simulink model "MCVT_Integration"  (R2020a)
settings: Solver=ode8  StartTime=0.0  StopTime=T_end  FixedStep=dt  RelTol=1e-3  EnableMultiTasking=on
values from neighbouring files (symbols this model uses):
  assumed_power_voltage = 1.25e2    (= 125)    [MCVT_Input_File.m]    (also in MCVT_Run_QuickCheck.m)
  battery_power_toggle = 1    [MCVT_Input_File.m]    (also in MCVT_Run_QuickCheck.m)
  battery_trickle_charge_param = 0.1    [MCVT_Input_File.m]    (also in MCVT_Run_QuickCheck.m)
  battery_trickle_leakage_param = 0.01    [MCVT_Input_File.m]    (also in MCVT_Run_QuickCheck.m)
  damage_case_id = 3    [MCVT_Input_File.m]    (also in MCVT_Run_QuickCheck.m = 1)
  dt = 1/fs    (= 0.001)    [MCVT_Run_QuickCheck.m]    (also in MCVT_Sim_Set.m)
  dt_2 = 1/fs_2    (= 0.001)    [MCVT_Run_QuickCheck.m]    (also in MCVT_Sim_Set.m)
  dt_3 = 1/fs_3    (= 0.001)    [MCVT_Run_QuickCheck.m]    (also in MCVT_Sim_Set.m)
  dt_5 = 1/fs_5    (= 0.005)    [MCVT_Run_QuickCheck.m]    (also in MCVT_Sim_Set.m)
  dt_8 = 1/fs_8    (= 0.05)    [MCVT_Run_QuickCheck.m]    (also in MCVT_Sim_Set.m)
  dt_9 = 1/fs_9    (= 0.001)    [MCVT_Run_QuickCheck.m]    (also in MCVT_Sim_Set.m)
  dust_met_duration = 3    [MCVT_Input_File.m]    (also in MCVT_Run_QuickCheck.m = 2)
  ECLSS_indoor_set_temperature = 298.15    [MCVT_Input_File.m]    (also in MCVT_Run_QuickCheck.m)
  energy_store_max_o = 150    [MCVT_Input_File.m]    (also in MCVT_Run_QuickCheck.m)
  energy_store_o = 120    [MCVT_Input_File.m]    (also in MCVT_Run_QuickCheck.m)
  fs = 1000    [MCVT_Run_QuickCheck.m]    (also in MCVT_Sim_Set.m)
  gain_charge_param = 1    [MCVT_Input_File.m]    (also in MCVT_Run_QuickCheck.m)
  ground_temperature = 373    [MCVT_Habitat_Design_Input_File.m]    (also in MCVT_Run_QuickCheck.m)
  I_max_charge = 800    [MCVT_Input_File.m]    (also in MCVT_Run_QuickCheck.m)
  I_max_discharge = 800    [MCVT_Input_File.m]    (also in MCVT_Run_QuickCheck.m)
  initial_pressure = 1e5    (= 100000)    [MCVT_Input_File.m]    (also in MCVT_Run_QuickCheck.m)
  initial_temperature = 295    [MCVT_Input_File.m]    (also in MCVT_Run_QuickCheck.m)
  interior_leak_rate = 0.00415    [MCVT_Input_File.m]    (also in MCVT_Run_QuickCheck.m)
  launch_dust_accum_start_time = 10    [MCVT_Input_File.m]    (also in MCVT_Run_QuickCheck.m = 2)
  launch_dust_accum_stop_time = 12    [MCVT_Input_File.m]    (also in MCVT_Run_QuickCheck.m = 3)
  meteorite_impact_time = 2    [MCVT_Input_File.m]    (also in MCVT_Run_QuickCheck.m = 1)
  nuclear_power_toggle = 1    [MCVT_Input_File.m]    (also in MCVT_Run_QuickCheck.m)
  print_interval = 30    [MCVT_Input_File.m]
  SOC_lower_thres = 0.5    [MCVT_Input_File.m]    (also in MCVT_Run_QuickCheck.m)
  SOC_upper_thres = 0.9    [MCVT_Input_File.m]    (also in MCVT_Run_QuickCheck.m)
  solar_power_toggle = 1    [MCVT_Input_File.m]    (also in MCVT_Run_QuickCheck.m)
  space_temperature = 4    [MCVT_Habitat_Design_Input_File.m]    (also in MCVT_Run_QuickCheck.m)
  Start_angle = 90    [MCVT_Input_File.m]    (also in MCVT_Run_QuickCheck.m)
undefined symbols (not defined in the file or in its folder): A_flow_restrictor, ACTIVITY_LOOKUP_TABLE, air, Aref, Bmap, Bmap2, Bmap3, comms, DiscIntNeverNeededParam, DmgSPL, dust_parameters, ECLSS_dust_damage_repair_time, ECLSS_heater_capacity, ECLSS_IPS_restrictor, ECLSS_IPS_setpointPressure, ECLSS_paint_damage_repair_time, ECLSS_radiatorLoop_discreteNumber, ECLSS_Vst, emptyActivity, expected_heat_add, FI, habitat, HIEM2SMM, HIEM2STM, HIEMDamageElements, impact_numbering, initial_SPLT_damage_index, input_air, input_Cond, input_CP, input_discretization, input_EV, input_Evap, input_radiatorLoop, K_ECLSS_dust_single, K_ECLSS_paint_single, K_Structure_damage_single, Kref, kW_to_W, meteoroidDustDep, mFRF, ndelays, nominalDustDep, noverlap, nuclear_toggle_gain, output_limits, panel_paint0, Ps0, reference_temperature, RepairTime_ECLSS_factor, Sensor_Location_SMM, SMM_T0, SMM_T1, SMM_T2, SMM_T3, SMM_T4, SMM_T4_0, SMM_T4_1, SMM_T4_2, solar_FDD_K ... (+73)
libraries: neural x350, simulink x39, xpcutilitieslib x7, xpcrtudplib x7, dspstat3 x1
(part 36 of 43)

== system MCVT_Integration/Sys3 - Power System/Power Generation Subsystem1/Nuclear Power Generation/SP-100 Power Generation/	Power Generation/Sensor Models/Thermocouple Model Stub/Omega XMO S-type Thermocouple ==
  Actual Temperature : Inport
  Kelvin to Celcuis : Bias  Bias=-273.15
  Thermocouple model 7 : Lookup_n-D  NumberOfTableDimensions=1  BreakpointsForDimension1=TypeS_degC  BeginIndexSearchUsingPreviousIndexResult=on  Table=TypeS_Volts  DiagnosticForOutOfRangeInput=Warning  InputSameDT=on
      settings: AttributesFormatString=%<bp1> to\n%<tableData>
  microvolts : Outport
  wires:
  Kelvin to Celcuis/1 -> Thermocouple model 7/1
  Actual Temperature/1 -> Kelvin to Celcuis/1
  Thermocouple model 7/1 -> microvolts/1

== system MCVT_Integration/Sys3 - Power System/Power Generation Subsystem1/Nuclear Power Generation/SP-100 Power Generation/	Power Generation/Sensor Models/Thermocouple Model2 Stub/MAX31856 Precision Thermocouple to Digital Converter with Linearization  ==
  Thermocouple Measurement : Inport
  External Cold Junction Measurement : Inport  Port=2
  Celcuis to Kelvin : Bias  Bias=273.15
  Thermocouple conditioning 7 : Lookup_n-D  NumberOfTableDimensions=1  BreakpointsForDimension1=TypeS_Volts  BeginIndexSearchUsingPreviousIndexResult=on  Table=TypeS_degC  DiagnosticForOutOfRangeInput=Warning  InputSameDT=on
      settings: AttributesFormatString=%<bp1> to\n%<tableData>
  Estimated Process Temperature : Outport
  Cyber Output : Outport  Port=2
  wires:
  Thermocouple conditioning 7/1 -> Celcuis to Kelvin/1
  Thermocouple Measurement/1 -> Thermocouple conditioning 7/1
  Celcuis to Kelvin/1 -> Estimated Process Temperature/1

== system MCVT_Integration/Sys3 - Power System/Power Generation Subsystem1/Nuclear Power Generation/SP-100 Power Generation/	Power Generation/Sensor Models/Thermocouple Model2 Stub/Omega XMO S-type Thermocouple ==
  Actual Temperature : Inport
  Kelvin to Celcuis : Bias  Bias=-273.15
  Thermocouple model 7 : Lookup_n-D  NumberOfTableDimensions=1  BreakpointsForDimension1=TypeS_degC  BeginIndexSearchUsingPreviousIndexResult=on  Table=TypeS_Volts  DiagnosticForOutOfRangeInput=Warning  InputSameDT=on
      settings: AttributesFormatString=%<bp1> to\n%<tableData>
  microvolts : Outport
  wires:
  Kelvin to Celcuis/1 -> Thermocouple model 7/1  "temp_test"
  Actual Temperature/1 -> Kelvin to Celcuis/1
  Thermocouple model 7/1 -> microvolts/1

== system MCVT_Integration/Sys3 - Power System/Power Generation Subsystem1/Nuclear Power Generation/SP-100 Power Generation/	Power Generation/Sensor Models/Thermocouple Model3 Stub/MAX31856 Precision Thermocouple to Digital Converter with Linearization  ==
  Thermocouple Measurement : Inport
  External Cold Junction Measurement : Inport  Port=2
  Celcuis to Kelvin : Bias  Bias=273.15
  Thermocouple conditioning 7 : Lookup_n-D  NumberOfTableDimensions=1  BreakpointsForDimension1=TypeS_Volts  BeginIndexSearchUsingPreviousIndexResult=on  Table=TypeS_degC  DiagnosticForOutOfRangeInput=Warning  InputSameDT=on
      settings: AttributesFormatString=%<bp1> to\n%<tableData>
  Estimated Process Temperature : Outport
  Cyber Output : Outport  Port=2
  wires:
  Thermocouple Measurement/1 -> Thermocouple conditioning 7/1
  Thermocouple conditioning 7/1 -> Celcuis to Kelvin/1
  Celcuis to Kelvin/1 -> Estimated Process Temperature/1

== system MCVT_Integration/Sys3 - Power System/Power Generation Subsystem1/Nuclear Power Generation/SP-100 Power Generation/	Power Generation/Sensor Models/Thermocouple Model3 Stub/Omega XMO S-type Thermocouple ==
  Actual Temperature : Inport
  Kelvin to Celcuis : Bias  Bias=-273.15
  Thermocouple model 7 : Lookup_n-D  NumberOfTableDimensions=1  BreakpointsForDimension1=TypeS_degC  BeginIndexSearchUsingPreviousIndexResult=on  Table=TypeS_Volts  DiagnosticForOutOfRangeInput=Warning  InputSameDT=on
      settings: AttributesFormatString=%<bp1> to\n%<tableData>
  microvolts : Outport
  wires:
  Kelvin to Celcuis/1 -> Thermocouple model 7/1
  Actual Temperature/1 -> Kelvin to Celcuis/1
  Thermocouple model 7/1 -> microvolts/1

== system MCVT_Integration/Sys3 - Power System/Power Generation Subsystem1/Nuclear Power Generation/SP-100 Power Generation/	Power Generation/Sensor Models/Thermocouple Model4 Stub/MAX31856 Precision Thermocouple to Digital Converter with Linearization  ==
  Thermocouple Measurement : Inport
  External Cold Junction Measurement : Inport  Port=2
  Celcuis to Kelvin : Bias  Bias=273.15
  Thermocouple conditioning 7 : Lookup_n-D  NumberOfTableDimensions=1  BreakpointsForDimension1=TypeS_Volts  BeginIndexSearchUsingPreviousIndexResult=on  Table=TypeS_degC  DiagnosticForOutOfRangeInput=Warning  InputSameDT=on
      settings: AttributesFormatString=%<bp1> to\n%<tableData>
  Estimated Process Temperature : Outport
  Cyber Output : Outport  Port=2
  wires:
  Thermocouple conditioning 7/1 -> Celcuis to Kelvin/1
  Thermocouple Measurement/1 -> Thermocouple conditioning 7/1
  Celcuis to Kelvin/1 -> Estimated Process Temperature/1

== system MCVT_Integration/Sys3 - Power System/Power Generation Subsystem1/Nuclear Power Generation/SP-100 Power Generation/	Power Generation/Sensor Models/Thermocouple Model4 Stub/Omega XMO S-type Thermocouple ==
  Actual Temperature : Inport
  Kelvin to Celcuis : Bias  Bias=-273.15
  Thermocouple model 7 : Lookup_n-D  NumberOfTableDimensions=1  BreakpointsForDimension1=TypeS_degC  BeginIndexSearchUsingPreviousIndexResult=on  Table=TypeS_Volts  DiagnosticForOutOfRangeInput=Warning  InputSameDT=on
      settings: AttributesFormatString=%<bp1> to\n%<tableData>
  microvolts : Outport
  wires:
  Kelvin to Celcuis/1 -> Thermocouple model 7/1
  Actual Temperature/1 -> Kelvin to Celcuis/1
  Thermocouple model 7/1 -> microvolts/1

== system MCVT_Integration/Sys3 - Power System/Power Generation Subsystem1/Nuclear Power Generation/SP-100 Power Generation/FDD Iterative Online Residual Analysis1/ Digital Twin of SP100/Residual Generation/Compare to Nominal ==
  Physical Output SP100 : Inport
  power nominal : Inport  Port=2
  Sub3_In_Intervention : Inport  Port=3
  CUSUM5 : Subsystem
  Mux5 : Mux  Inputs=3
  Subtract10 : Sum  Inputs=-+
  Terminator5 : Terminator
  sub3_cusum_sigma : Constant  Value=sub3_cusum_sigma
  FDD : Outport
  wires:
  [nom_power] -> Subtract10/2  "power_nominal (kW)"
  Physical Output SP100/1 -> [pow_actual]  "pow_actual (kW)"
  Subtract10/1 -> [power_resids]  "Power Residuals Compare"
  [sigma] -> CUSUM5/2(sigma)
  CUSUM5/1(Bias Indication) -> Mux5/1, [FDD_signal]  "Pow_bias"
  CUSUM5/2(Positive Bias Indication) -> Mux5/2  "Pow_Pos_bias"
  CUSUM5/3(Negative Bias Indication) -> Mux5/3  "Pow_Neg_bias"
  Mux5/1 -> Terminator5/1  "Power_indicators_compare"
  [fdd_int] -> CUSUM5/3(Sub3_In_Intervention)
  [FDD_signal] -> FDD/1
  [pow_actual] -> Subtract10/1
  power nominal/1 -> [nom_power]  "power_nominal (kW)"
  Sub3_In_Intervention/1 -> [fdd_int]
  [power_resids] -> CUSUM5/1(residual)
  sub3_cusum_sigma/1 -> [sigma]

== system MCVT_Integration/Sys3 - Power System/Power Generation Subsystem1/Nuclear Power Generation/SP-100 Power Generation/	Power Generation/Nominal Power/Sensor Models/Cold Junction Temperature Sensing Model/ Resistance Temperature Detector ==
  Physical Interdependency - IN : Inport
  Switch Control : Inport  Port=2
  R = U/I : Gain  Gain=1/0.001
  R vs T : MATLAB Function  TreatAsAtomicUnit=on
  RTD with Self-heat Error : Subsystem
  RTD without Self-heat Error : Subsystem
  Self-heat Calculation : Subsystem
  Switch ON/OFF Control Block : Subsystem
  T(Celcuis) vs R : MATLAB Function  TreatAsAtomicUnit=on
  Read Temperature : Outport  VectorParamsAs1DForOutWhenUnconnected=off
  Measured Temperature : Outport  Port=2  VectorParamsAs1DForOutWhenUnconnected=off
  wires:
  T(Celcuis) vs R/1(R) -> RTD without Self-heat Error/1(Resistance Change without Error), Self-heat Calculation/2(Resistance Change without Error)
  R vs T/1(T) -> Measured Temperature/1
  RTD with Self-heat Error/1(Voltage Output with Error) -> R = U/I/1
  Self-heat Calculation/1(Resistance Change with Error) -> RTD with Self-heat Error/1(Resistance Change with Error)
  R = U/I/1 -> R vs T/1(R)
  RTD without Self-heat Error/1(Current in Circuit) -> Self-heat Calculation/3(Current in Circuit)
  Switch ON/OFF Control Block/1(Out1) -> T(Celcuis) vs R/1(T), Self-heat Calculation/1(Real Temperature)
  Switch Control/1 -> Switch ON/OFF Control Block/1(In1)
  Physical Interdependency - IN/1 -> Read Temperature/1, Switch ON/OFF Control Block/2(In2)

== system MCVT_Integration/Sys3 - Power System/Power Generation Subsystem1/Nuclear Power Generation/SP-100 Power Generation/	Power Generation/Nominal Power/Sensor Models/Cold Junction Temperature Sensing Model1/ Resistance Temperature Detector ==
  Physical Interdependency - IN : Inport
  Switch Control : Inport  Port=2
  R = U/I : Gain  Gain=1/0.001
  R vs T : MATLAB Function  TreatAsAtomicUnit=on
  RTD with Self-heat Error : Subsystem
  RTD without Self-heat Error : Subsystem
  Self-heat Calculation : Subsystem
  Switch ON/OFF Control Block : Subsystem
  T(Celcuis) vs R : MATLAB Function  TreatAsAtomicUnit=on
  Temperature Output with Error : Scope
  Read Temperature : Outport  VectorParamsAs1DForOutWhenUnconnected=off
  Measured Temperature : Outport  Port=2  VectorParamsAs1DForOutWhenUnconnected=off
  wires:
  T(Celcuis) vs R/1(R) -> RTD without Self-heat Error/1(Resistance Change without Error), Self-heat Calculation/2(Resistance Change without Error)
  R vs T/1(T) -> Measured Temperature/1, Temperature Output with Error/1
  RTD with Self-heat Error/1(Voltage Output with Error) -> R = U/I/1
  Self-heat Calculation/1(Resistance Change with Error) -> RTD with Self-heat Error/1(Resistance Change with Error)
  R = U/I/1 -> R vs T/1(R)
  RTD without Self-heat Error/1(Current in Circuit) -> Self-heat Calculation/3(Current in Circuit)
  Switch ON/OFF Control Block/1(Out1) -> T(Celcuis) vs R/1(T), Self-heat Calculation/1(Real Temperature)
  Switch Control/1 -> Switch ON/OFF Control Block/1(In1)
  Physical Interdependency - IN/1 -> Read Temperature/1, Switch ON/OFF Control Block/2(In2)

== system MCVT_Integration/Sys3 - Power System/Power Generation Subsystem1/Nuclear Power Generation/SP-100 Power Generation/	Power Generation/Nominal Power/Sensor Models/Cold Junction Temperature Sensing Model2/ Resistance Temperature Detector ==
  Physical Interdependency - IN : Inport
  Switch Control : Inport  Port=2
  R = U/I : Gain  Gain=1/0.001
  R vs T : MATLAB Function  TreatAsAtomicUnit=on
  RTD with Self-heat Error : Subsystem
  RTD without Self-heat Error : Subsystem
  Self-heat Calculation : Subsystem
  Switch ON/OFF Control Block : Subsystem
  T(Celcuis) vs R : MATLAB Function  TreatAsAtomicUnit=on
  Temperature Output with Error : Scope
  Read Temperature : Outport  VectorParamsAs1DForOutWhenUnconnected=off
  Measured Temperature : Outport  Port=2  VectorParamsAs1DForOutWhenUnconnected=off
  wires:
  Physical Interdependency - IN/1 -> Switch ON/OFF Control Block/2(In2), Read Temperature/1
  Switch Control/1 -> Switch ON/OFF Control Block/1(In1)
  Switch ON/OFF Control Block/1(Out1) -> Self-heat Calculation/1(Real Temperature), T(Celcuis) vs R/1(T)
  RTD without Self-heat Error/1(Current in Circuit) -> Self-heat Calculation/3(Current in Circuit)
  R = U/I/1 -> R vs T/1(R)
  Self-heat Calculation/1(Resistance Change with Error) -> RTD with Self-heat Error/1(Resistance Change with Error)
  RTD with Self-heat Error/1(Voltage Output with Error) -> R = U/I/1
  R vs T/1(T) -> Temperature Output with Error/1, Measured Temperature/1
  T(Celcuis) vs R/1(R) -> Self-heat Calculation/2(Resistance Change without Error), RTD without Self-heat Error/1(Resistance Change without Error)

== system MCVT_Integration/Sys3 - Power System/Power Generation Subsystem1/Nuclear Power Generation/SP-100 Power Generation/	Power Generation/Nominal Power/Sensor Models/Cold Junction Temperature Sensing Model3/ Resistance Temperature Detector ==
  Physical Interdependency - IN : Inport
  Switch Control : Inport  Port=2
  R = U/I : Gain  Gain=1/0.001
  R vs T : MATLAB Function  TreatAsAtomicUnit=on
  RTD with Self-heat Error : Subsystem
  RTD without Self-heat Error : Subsystem
  Self-heat Calculation : Subsystem
  Switch ON/OFF Control Block : Subsystem
  T(Celcuis) vs R : MATLAB Function  TreatAsAtomicUnit=on
  Temperature Output with Error : Scope
  Read Temperature : Outport  VectorParamsAs1DForOutWhenUnconnected=off
  Measured Temperature : Outport  Port=2  VectorParamsAs1DForOutWhenUnconnected=off
  wires:
  T(Celcuis) vs R/1(R) -> RTD without Self-heat Error/1(Resistance Change without Error), Self-heat Calculation/2(Resistance Change without Error)
  R vs T/1(T) -> Measured Temperature/1, Temperature Output with Error/1
  RTD with Self-heat Error/1(Voltage Output with Error) -> R = U/I/1
  Self-heat Calculation/1(Resistance Change with Error) -> RTD with Self-heat Error/1(Resistance Change with Error)
  R = U/I/1 -> R vs T/1(R)
  RTD without Self-heat Error/1(Current in Circuit) -> Self-heat Calculation/3(Current in Circuit)
  Switch ON/OFF Control Block/1(Out1) -> T(Celcuis) vs R/1(T), Self-heat Calculation/1(Real Temperature)
  Switch Control/1 -> Switch ON/OFF Control Block/1(In1)
  Physical Interdependency - IN/1 -> Read Temperature/1, Switch ON/OFF Control Block/2(In2)

== system MCVT_Integration/Sys3 - Power System/Power Generation Subsystem1/Nuclear Power Generation/SP-100 Power Generation/	Power Generation/Nominal Power/Sensor Models/Cold Junction Temperature Sensing Model4/ Resistance Temperature Detector ==
  Physical Interdependency - IN : Inport
  Switch Control : Inport  Port=2
  R = U/I : Gain  Gain=1/0.001
  R vs T : MATLAB Function  TreatAsAtomicUnit=on
  RTD with Self-heat Error : Subsystem
  RTD without Self-heat Error : Subsystem
  Self-heat Calculation : Subsystem
  Switch ON/OFF Control Block : Subsystem
  T(Celcuis) vs R : MATLAB Function  TreatAsAtomicUnit=on
  Temperature Output with Error : Scope
  Read Temperature : Outport  VectorParamsAs1DForOutWhenUnconnected=off
  Measured Temperature : Outport  Port=2  VectorParamsAs1DForOutWhenUnconnected=off
  wires:
  Physical Interdependency - IN/1 -> Switch ON/OFF Control Block/2(In2), Read Temperature/1
  Switch Control/1 -> Switch ON/OFF Control Block/1(In1)
  Switch ON/OFF Control Block/1(Out1) -> Self-heat Calculation/1(Real Temperature), T(Celcuis) vs R/1(T)
  RTD without Self-heat Error/1(Current in Circuit) -> Self-heat Calculation/3(Current in Circuit)
  R = U/I/1 -> R vs T/1(R)
  Self-heat Calculation/1(Resistance Change with Error) -> RTD with Self-heat Error/1(Resistance Change with Error)
  RTD with Self-heat Error/1(Voltage Output with Error) -> R = U/I/1
  R vs T/1(T) -> Temperature Output with Error/1, Measured Temperature/1
  T(Celcuis) vs R/1(R) -> Self-heat Calculation/2(Resistance Change without Error), RTD without Self-heat Error/1(Resistance Change without Error)

== system MCVT_Integration/Sys3 - Power System/Power Generation Subsystem1/Nuclear Power Generation/SP-100 Power Generation/	Power Generation/Nominal Power/Sensor Models/Thermocouple Mode Stub/MAX31856 Precision Thermocouple to Digital Converter with Linearization  ==
  Thermocouple Measurement : Inport
  External Cold Junction Measurement : Inport  Port=2
  Celcuis to Kelvin : Bias  Bias=273.15
  Thermocouple conditioning 7 : Lookup_n-D  NumberOfTableDimensions=1  BreakpointsForDimension1=TypeS_Volts  BeginIndexSearchUsingPreviousIndexResult=on  Table=TypeS_degC  DiagnosticForOutOfRangeInput=Warning  InputSameDT=on
      settings: AttributesFormatString=%<bp1> to\n%<tableData>
  Estimated Process Temperature : Outport
  Cyber Output : Outport  Port=2
  wires:
  Thermocouple conditioning 7/1 -> Celcuis to Kelvin/1
  Thermocouple Measurement/1 -> Thermocouple conditioning 7/1
  Celcuis to Kelvin/1 -> Estimated Process Temperature/1

== system MCVT_Integration/Sys3 - Power System/Power Generation Subsystem1/Nuclear Power Generation/SP-100 Power Generation/	Power Generation/Nominal Power/Sensor Models/Thermocouple Mode Stub/Omega XMO S-type Thermocouple ==
  Actual Temperature : Inport
  Kelvin to Celcuis : Bias  Bias=-273.15
  Thermocouple model 7 : Lookup_n-D  NumberOfTableDimensions=1  BreakpointsForDimension1=TypeS_degC  BeginIndexSearchUsingPreviousIndexResult=on  Table=TypeS_Volts  DiagnosticForOutOfRangeInput=Warning  InputSameDT=on
      settings: AttributesFormatString=%<bp1> to\n%<tableData>
  microvolts : Outport
  wires:
  Actual Temperature/1 -> Kelvin to Celcuis/1
  Kelvin to Celcuis/1 -> Thermocouple model 7/1
  Thermocouple model 7/1 -> microvolts/1

== system MCVT_Integration/Sys3 - Power System/Power Generation Subsystem1/Nuclear Power Generation/SP-100 Power Generation/	Power Generation/Nominal Power/Sensor Models/Thermocouple Model Stub/MAX31856 Precision Thermocouple to Digital Converter with Linearization  ==
  Thermocouple Measurement : Inport
  External Cold Junction Measurement : Inport  Port=2
  Celcuis to Kelvin : Bias  Bias=273.15
  Thermocouple conditioning 7 : Lookup_n-D  NumberOfTableDimensions=1  BreakpointsForDimension1=TypeS_Volts  BeginIndexSearchUsingPreviousIndexResult=on  Table=TypeS_degC  DiagnosticForOutOfRangeInput=Warning  InputSameDT=on
      settings: AttributesFormatString=%<bp1> to\n%<tableData>
  Estimated Process Temperature : Outport
  Cyber Output : Outport  Port=2
  wires:
  Thermocouple conditioning 7/1 -> Celcuis to Kelvin/1
  Thermocouple Measurement/1 -> Thermocouple conditioning 7/1
  Celcuis to Kelvin/1 -> Estimated Process Temperature/1

== system MCVT_Integration/Sys3 - Power System/Power Generation Subsystem1/Nuclear Power Generation/SP-100 Power Generation/	Power Generation/Nominal Power/Sensor Models/Thermocouple Model Stub/Omega XMO S-type Thermocouple ==
  Actual Temperature : Inport
  Kelvin to Celcuis : Bias  Bias=-273.15
  Thermocouple model 7 : Lookup_n-D  NumberOfTableDimensions=1  BreakpointsForDimension1=TypeS_degC  BeginIndexSearchUsingPreviousIndexResult=on  Table=TypeS_Volts  DiagnosticForOutOfRangeInput=Warning  InputSameDT=on
      settings: AttributesFormatString=%<bp1> to\n%<tableData>
  microvolts : Outport
  wires:
  Kelvin to Celcuis/1 -> Thermocouple model 7/1
  Actual Temperature/1 -> Kelvin to Celcuis/1
  Thermocouple model 7/1 -> microvolts/1

== system MCVT_Integration/Sys3 - Power System/Power Generation Subsystem1/Nuclear Power Generation/SP-100 Power Generation/	Power Generation/Nominal Power/Sensor Models/Thermocouple Model2 Stub/MAX31856 Precision Thermocouple to Digital Converter with Linearization  ==
  Thermocouple Measurement : Inport
  External Cold Junction Measurement : Inport  Port=2
  Celcuis to Kelvin : Bias  Bias=273.15
  Thermocouple conditioning 7 : Lookup_n-D  NumberOfTableDimensions=1  BreakpointsForDimension1=TypeS_Volts  BeginIndexSearchUsingPreviousIndexResult=on  Table=TypeS_degC  DiagnosticForOutOfRangeInput=Warning  InputSameDT=on
      settings: AttributesFormatString=%<bp1> to\n%<tableData>
  Estimated Process Temperature : Outport
  Cyber Output : Outport  Port=2
  wires:
  Thermocouple conditioning 7/1 -> Celcuis to Kelvin/1
  Thermocouple Measurement/1 -> Thermocouple conditioning 7/1
  Celcuis to Kelvin/1 -> Estimated Process Temperature/1

== system MCVT_Integration/Sys3 - Power System/Power Generation Subsystem1/Nuclear Power Generation/SP-100 Power Generation/	Power Generation/Nominal Power/Sensor Models/Thermocouple Model2 Stub/Omega XMO S-type Thermocouple ==
  Actual Temperature : Inport
  Kelvin to Celcuis : Bias  Bias=-273.15
  Thermocouple model 7 : Lookup_n-D  NumberOfTableDimensions=1  BreakpointsForDimension1=TypeS_degC  BeginIndexSearchUsingPreviousIndexResult=on  Table=TypeS_Volts  DiagnosticForOutOfRangeInput=Warning  InputSameDT=on
      settings: AttributesFormatString=%<bp1> to\n%<tableData>
  microvolts : Outport
  wires:
  Kelvin to Celcuis/1 -> Thermocouple model 7/1  "temp_test"
  Actual Temperature/1 -> Kelvin to Celcuis/1
  Thermocouple model 7/1 -> microvolts/1

== system MCVT_Integration/Sys3 - Power System/Power Generation Subsystem1/Nuclear Power Generation/SP-100 Power Generation/	Power Generation/Nominal Power/Sensor Models/Thermocouple Model3 Stub/MAX31856 Precision Thermocouple to Digital Converter with Linearization  ==
  Thermocouple Measurement : Inport
  External Cold Junction Measurement : Inport  Port=2
  Celcuis to Kelvin : Bias  Bias=273.15
  Thermocouple conditioning 7 : Lookup_n-D  NumberOfTableDimensions=1  BreakpointsForDimension1=TypeS_Volts  BeginIndexSearchUsingPreviousIndexResult=on  Table=TypeS_degC  DiagnosticForOutOfRangeInput=Warning  InputSameDT=on
      settings: AttributesFormatString=%<bp1> to\n%<tableData>
  Estimated Process Temperature : Outport
  Cyber Output : Outport  Port=2
  wires:
  Thermocouple Measurement/1 -> Thermocouple conditioning 7/1
  Thermocouple conditioning 7/1 -> Celcuis to Kelvin/1
  Celcuis to Kelvin/1 -> Estimated Process Temperature/1

== system MCVT_Integration/Sys3 - Power System/Power Generation Subsystem1/Nuclear Power Generation/SP-100 Power Generation/	Power Generation/Nominal Power/Sensor Models/Thermocouple Model3 Stub/Omega XMO S-type Thermocouple ==
  Actual Temperature : Inport
  Kelvin to Celcuis : Bias  Bias=-273.15
  Thermocouple model 7 : Lookup_n-D  NumberOfTableDimensions=1  BreakpointsForDimension1=TypeS_degC  BeginIndexSearchUsingPreviousIndexResult=on  Table=TypeS_Volts  DiagnosticForOutOfRangeInput=Warning  InputSameDT=on
      settings: AttributesFormatString=%<bp1> to\n%<tableData>
  microvolts : Outport
  wires:
  Kelvin to Celcuis/1 -> Thermocouple model 7/1
  Actual Temperature/1 -> Kelvin to Celcuis/1
  Thermocouple model 7/1 -> microvolts/1

== system MCVT_Integration/Sys3 - Power System/Power Generation Subsystem1/Nuclear Power Generation/SP-100 Power Generation/	Power Generation/Nominal Power/Sensor Models/Thermocouple Model4 Stub/MAX31856 Precision Thermocouple to Digital Converter with Linearization  ==
  Thermocouple Measurement : Inport
  External Cold Junction Measurement : Inport  Port=2
  Celcuis to Kelvin : Bias  Bias=273.15
  Thermocouple conditioning 7 : Lookup_n-D  NumberOfTableDimensions=1  BreakpointsForDimension1=TypeS_Volts  BeginIndexSearchUsingPreviousIndexResult=on  Table=TypeS_degC  DiagnosticForOutOfRangeInput=Warning  InputSameDT=on
      settings: AttributesFormatString=%<bp1> to\n%<tableData>
  Estimated Process Temperature : Outport
  Cyber Output : Outport  Port=2
  wires:
  Thermocouple conditioning 7/1 -> Celcuis to Kelvin/1
  Thermocouple Measurement/1 -> Thermocouple conditioning 7/1
  Celcuis to Kelvin/1 -> Estimated Process Temperature/1

== system MCVT_Integration/Sys3 - Power System/Power Generation Subsystem1/Nuclear Power Generation/SP-100 Power Generation/	Power Generation/Nominal Power/Sensor Models/Thermocouple Model4 Stub/Omega XMO S-type Thermocouple ==
  Actual Temperature : Inport
  Kelvin to Celcuis : Bias  Bias=-273.15
  Thermocouple model 7 : Lookup_n-D  NumberOfTableDimensions=1  BreakpointsForDimension1=TypeS_degC  BeginIndexSearchUsingPreviousIndexResult=on  Table=TypeS_Volts  DiagnosticForOutOfRangeInput=Warning  InputSameDT=on
      settings: AttributesFormatString=%<bp1> to\n%<tableData>
  microvolts : Outport
  wires:
  Kelvin to Celcuis/1 -> Thermocouple model 7/1
  Actual Temperature/1 -> Kelvin to Celcuis/1
  Thermocouple model 7/1 -> microvolts/1

== system MCVT_Integration/Sys3 - Power System/Power Generation Subsystem1/Nuclear Power Generation/SP-100 Power Generation/	Power Generation/Sensor Models/Cold Junction Temperature Sensing Model/ Resistance Temperature Detector/RTD with Self-heat Error ==
  Resistance Change with Error : Inport
  " Current in Circuit" : Constant  Value=0.001
  MATLAB Function : MATLAB Function  TreatAsAtomicUnit=on
  Voltage Output with Error : Outport  VectorParamsAs1DForOutWhenUnconnected=off
  wires:
  Resistance Change with Error/1 -> MATLAB Function/2(R)
  MATLAB Function/1(U) -> Voltage Output with Error/1
  " Current in Circuit"/1 -> MATLAB Function/1(I)

== system MCVT_Integration/Sys3 - Power System/Power Generation Subsystem1/Nuclear Power Generation/SP-100 Power Generation/	Power Generation/Sensor Models/Cold Junction Temperature Sensing Model/ Resistance Temperature Detector/RTD without Self-heat Error ==
  Resistance Change without Error : Inport
  " Current in Circuit" : Constant  Value=0.001
  MATLAB Function : MATLAB Function  TreatAsAtomicUnit=on
  Current in Circuit : Outport  VectorParamsAs1DForOutWhenUnconnected=off
  Voltage Output without Error : Outport  Port=2  VectorParamsAs1DForOutWhenUnconnected=off
  wires:
  Resistance Change without Error/1 -> MATLAB Function/2(R)
  MATLAB Function/2(U) -> Voltage Output without Error/1
  MATLAB Function/1(I) -> Current in Circuit/1
  " Current in Circuit"/1 -> MATLAB Function/1("I ")

== system MCVT_Integration/Sys3 - Power System/Power Generation Subsystem1/Nuclear Power Generation/SP-100 Power Generation/	Power Generation/Sensor Models/Cold Junction Temperature Sensing Model/ Resistance Temperature Detector/Self-heat Calculation ==
  note: Power = I 2 *R
  Real Temperature : Inport
  Resistance Change without Error : Inport  Port=2
  Current in Circuit : Inport  Port=3
  "  T vs R " : MATLAB Function  TreatAsAtomicUnit=on
  Add1 : Sum
  Divide1 : Product  Inputs=*/
  Gain : Gain  Gain=1000
  Product : Product
  Self Heating Constant (mW/℃) : Constant  Value=15
  Square : Math  Operator=square  SignedPower=on  SaturateOnIntegerOverflow=on
  Resistance Change with Error : Outport  VectorParamsAs1DForOutWhenUnconnected=off
  Self-heat Error : Outport  Port=2  VectorParamsAs1DForOutWhenUnconnected=off
  wires:
  Self Heating Constant (mW/℃)/1 -> Divide1/2
  Product/1 -> Divide1/1
  Add1/1 -> "  T vs R "/1(T)
  Divide1/1 -> Self-heat Error/1, Add1/2
  Resistance Change without Error/1 -> Product/1
  "  T vs R "/1(R) -> Resistance Change with Error/1
  Real Temperature/1 -> Add1/1
  Gain/1 -> Square/1
  Square/1 -> Product/2
  Current in Circuit/1 -> Gain/1

== system MCVT_Integration/Sys3 - Power System/Power Generation Subsystem1/Nuclear Power Generation/SP-100 Power Generation/	Power Generation/Sensor Models/Cold Junction Temperature Sensing Model/ Resistance Temperature Detector/Switch ON//OFF Control Block ==
  In1 : Inport
  In2 : Inport  Port=2
  Multiport Switch1 : MultiPortSwitch  Inputs=2
  Switch OFF : Subsystem
  Switch ON : Subsystem
  Out1 : Outport  VectorParamsAs1DForOutWhenUnconnected=off
  wires:
  Multiport Switch1/1 -> Out1/1
  In2/1 -> Switch ON/1(In1), Switch OFF/1(In1)
  In1/1 -> Multiport Switch1/1
  Switch ON/1(ON) -> Multiport Switch1/2
  Switch OFF/1(OFF) -> Multiport Switch1/3

== system MCVT_Integration/Sys3 - Power System/Power Generation Subsystem1/Nuclear Power Generation/SP-100 Power Generation/	Power Generation/Sensor Models/Cold Junction Temperature Sensing Model1/ Resistance Temperature Detector/RTD with Self-heat Error ==
  Resistance Change with Error : Inport
  " Current in Circuit" : Constant  Value=0.001
  MATLAB Function : MATLAB Function  TreatAsAtomicUnit=on
  Voltage Output with Error : Outport  VectorParamsAs1DForOutWhenUnconnected=off
  wires:
  Resistance Change with Error/1 -> MATLAB Function/2(R)
  MATLAB Function/1(U) -> Voltage Output with Error/1
  " Current in Circuit"/1 -> MATLAB Function/1(I)

== system MCVT_Integration/Sys3 - Power System/Power Generation Subsystem1/Nuclear Power Generation/SP-100 Power Generation/	Power Generation/Sensor Models/Cold Junction Temperature Sensing Model1/ Resistance Temperature Detector/RTD without Self-heat Error ==
  Resistance Change without Error : Inport
  " Current in Circuit" : Constant  Value=0.001
  MATLAB Function : MATLAB Function  TreatAsAtomicUnit=on
  Current in Circuit : Outport  VectorParamsAs1DForOutWhenUnconnected=off
  Voltage Output without Error : Outport  Port=2  VectorParamsAs1DForOutWhenUnconnected=off
  wires:
  Resistance Change without Error/1 -> MATLAB Function/2(R)
  MATLAB Function/2(U) -> Voltage Output without Error/1
  MATLAB Function/1(I) -> Current in Circuit/1
  " Current in Circuit"/1 -> MATLAB Function/1("I ")

== system MCVT_Integration/Sys3 - Power System/Power Generation Subsystem1/Nuclear Power Generation/SP-100 Power Generation/	Power Generation/Sensor Models/Cold Junction Temperature Sensing Model1/ Resistance Temperature Detector/Self-heat Calculation ==
  note: Power = I 2 *R
  Real Temperature : Inport
  Resistance Change without Error : Inport  Port=2
  Current in Circuit : Inport  Port=3
  "  T vs R " : MATLAB Function  TreatAsAtomicUnit=on
  Add1 : Sum
  Divide1 : Product  Inputs=*/
  Gain : Gain  Gain=1000
  Product : Product
  Self Heating Constant (mW/℃) : Constant  Value=15
  Square : Math  Operator=square  SignedPower=on  SaturateOnIntegerOverflow=on
  Resistance Change with Error : Outport  VectorParamsAs1DForOutWhenUnconnected=off
  Self-heat Error : Outport  Port=2  VectorParamsAs1DForOutWhenUnconnected=off
  wires:
  Self Heating Constant (mW/℃)/1 -> Divide1/2
  Product/1 -> Divide1/1
  Add1/1 -> "  T vs R "/1(T)
  Divide1/1 -> Self-heat Error/1, Add1/2  "self_heat_error"
  Resistance Change without Error/1 -> Product/1
  "  T vs R "/1(R) -> Resistance Change with Error/1
  Real Temperature/1 -> Add1/1
  Gain/1 -> Square/1
  Square/1 -> Product/2
  Current in Circuit/1 -> Gain/1

== system MCVT_Integration/Sys3 - Power System/Power Generation Subsystem1/Nuclear Power Generation/SP-100 Power Generation/	Power Generation/Sensor Models/Cold Junction Temperature Sensing Model1/ Resistance Temperature Detector/Switch ON//OFF Control Block ==
  In1 : Inport
  In2 : Inport  Port=2
  Multiport Switch1 : MultiPortSwitch  Inputs=2
  Switch OFF : Subsystem
  Switch ON : Subsystem
  Out1 : Outport  VectorParamsAs1DForOutWhenUnconnected=off
  wires:
  Multiport Switch1/1 -> Out1/1
  In2/1 -> Switch ON/1(In1), Switch OFF/1(In1)
  In1/1 -> Multiport Switch1/1
  Switch ON/1(ON) -> Multiport Switch1/2
  Switch OFF/1(OFF) -> Multiport Switch1/3

== system MCVT_Integration/Sys3 - Power System/Power Generation Subsystem1/Nuclear Power Generation/SP-100 Power Generation/	Power Generation/Sensor Models/Cold Junction Temperature Sensing Model2/ Resistance Temperature Detector/RTD with Self-heat Error ==
  Resistance Change with Error : Inport
  " Current in Circuit" : Constant  Value=0.001
  MATLAB Function : MATLAB Function  TreatAsAtomicUnit=on
  Voltage Output with Error : Outport  VectorParamsAs1DForOutWhenUnconnected=off
  wires:
  " Current in Circuit"/1 -> MATLAB Function/1(I)
  MATLAB Function/1(U) -> Voltage Output with Error/1
  Resistance Change with Error/1 -> MATLAB Function/2(R)

== system MCVT_Integration/Sys3 - Power System/Power Generation Subsystem1/Nuclear Power Generation/SP-100 Power Generation/	Power Generation/Sensor Models/Cold Junction Temperature Sensing Model2/ Resistance Temperature Detector/RTD without Self-heat Error ==
  Resistance Change without Error : Inport
  " Current in Circuit" : Constant  Value=0.001
  MATLAB Function : MATLAB Function  TreatAsAtomicUnit=on
  Current in Circuit : Outport  VectorParamsAs1DForOutWhenUnconnected=off
  Voltage Output without Error : Outport  Port=2  VectorParamsAs1DForOutWhenUnconnected=off
  wires:
  " Current in Circuit"/1 -> MATLAB Function/1("I ")
  MATLAB Function/1(I) -> Current in Circuit/1
  MATLAB Function/2(U) -> Voltage Output without Error/1
  Resistance Change without Error/1 -> MATLAB Function/2(R)

== system MCVT_Integration/Sys3 - Power System/Power Generation Subsystem1/Nuclear Power Generation/SP-100 Power Generation/	Power Generation/Sensor Models/Cold Junction Temperature Sensing Model2/ Resistance Temperature Detector/Self-heat Calculation ==
  note: Power = I 2 *R
  Real Temperature : Inport
  Resistance Change without Error : Inport  Port=2
  Current in Circuit : Inport  Port=3
  "  T vs R " : MATLAB Function  TreatAsAtomicUnit=on
  Add1 : Sum
  Divide1 : Product  Inputs=*/
  Gain : Gain  Gain=1000
  Product : Product
  Self Heating Constant (mW/℃) : Constant  Value=15
  Square : Math  Operator=square  SignedPower=on  SaturateOnIntegerOverflow=on
  Resistance Change with Error : Outport  VectorParamsAs1DForOutWhenUnconnected=off
  Self-heat Error : Outport  Port=2  VectorParamsAs1DForOutWhenUnconnected=off
  wires:
  Current in Circuit/1 -> Gain/1
  Square/1 -> Product/2
  Gain/1 -> Square/1
  Real Temperature/1 -> Add1/1
  "  T vs R "/1(R) -> Resistance Change with Error/1
  Resistance Change without Error/1 -> Product/1
  Divide1/1 -> Add1/2, Self-heat Error/1
  Add1/1 -> "  T vs R "/1(T)
  Product/1 -> Divide1/1
  Self Heating Constant (mW/℃)/1 -> Divide1/2

== system MCVT_Integration/Sys3 - Power System/Power Generation Subsystem1/Nuclear Power Generation/SP-100 Power Generation/	Power Generation/Sensor Models/Cold Junction Temperature Sensing Model2/ Resistance Temperature Detector/Switch ON//OFF Control Block ==
  In1 : Inport
  In2 : Inport  Port=2
  Multiport Switch1 : MultiPortSwitch  Inputs=2
  Switch OFF : Subsystem
  Switch ON : Subsystem
  Out1 : Outport  VectorParamsAs1DForOutWhenUnconnected=off
  wires:
  Switch OFF/1(OFF) -> Multiport Switch1/3
  Switch ON/1(ON) -> Multiport Switch1/2
  In1/1 -> Multiport Switch1/1
  In2/1 -> Switch OFF/1(In1), Switch ON/1(In1)
  Multiport Switch1/1 -> Out1/1

== system MCVT_Integration/Sys3 - Power System/Power Generation Subsystem1/Nuclear Power Generation/SP-100 Power Generation/	Power Generation/Sensor Models/Cold Junction Temperature Sensing Model3/ Resistance Temperature Detector/RTD with Self-heat Error ==
  Resistance Change with Error : Inport
  " Current in Circuit" : Constant  Value=0.001
  MATLAB Function : MATLAB Function  TreatAsAtomicUnit=on
  Voltage Output with Error : Outport  VectorParamsAs1DForOutWhenUnconnected=off
  wires:
  Resistance Change with Error/1 -> MATLAB Function/2(R)
  MATLAB Function/1(U) -> Voltage Output with Error/1
  " Current in Circuit"/1 -> MATLAB Function/1(I)

== system MCVT_Integration/Sys3 - Power System/Power Generation Subsystem1/Nuclear Power Generation/SP-100 Power Generation/	Power Generation/Sensor Models/Cold Junction Temperature Sensing Model3/ Resistance Temperature Detector/RTD without Self-heat Error ==
  Resistance Change without Error : Inport
  " Current in Circuit" : Constant  Value=0.001
  MATLAB Function : MATLAB Function  TreatAsAtomicUnit=on
  Current in Circuit : Outport  VectorParamsAs1DForOutWhenUnconnected=off
  Voltage Output without Error : Outport  Port=2  VectorParamsAs1DForOutWhenUnconnected=off
  wires:
  Resistance Change without Error/1 -> MATLAB Function/2(R)
  MATLAB Function/2(U) -> Voltage Output without Error/1
  MATLAB Function/1(I) -> Current in Circuit/1
  " Current in Circuit"/1 -> MATLAB Function/1("I ")

== system MCVT_Integration/Sys3 - Power System/Power Generation Subsystem1/Nuclear Power Generation/SP-100 Power Generation/	Power Generation/Sensor Models/Cold Junction Temperature Sensing Model3/ Resistance Temperature Detector/Self-heat Calculation ==
  note: Power = I 2 *R
  Real Temperature : Inport
  Resistance Change without Error : Inport  Port=2
  Current in Circuit : Inport  Port=3
  "  T vs R " : MATLAB Function  TreatAsAtomicUnit=on
  Add1 : Sum
  Divide1 : Product  Inputs=*/
  Gain : Gain  Gain=1000
  Product : Product
  Self Heating Constant (mW/℃) : Constant  Value=15
  Square : Math  Operator=square  SignedPower=on  SaturateOnIntegerOverflow=on
  Resistance Change with Error : Outport  VectorParamsAs1DForOutWhenUnconnected=off
  Self-heat Error : Outport  Port=2  VectorParamsAs1DForOutWhenUnconnected=off
  wires:
  Self Heating Constant (mW/℃)/1 -> Divide1/2
  Product/1 -> Divide1/1
  Add1/1 -> "  T vs R "/1(T)
  Divide1/1 -> Self-heat Error/1, Add1/2
  Resistance Change without Error/1 -> Product/1
  "  T vs R "/1(R) -> Resistance Change with Error/1
  Real Temperature/1 -> Add1/1
  Gain/1 -> Square/1
  Square/1 -> Product/2
  Current in Circuit/1 -> Gain/1

== system MCVT_Integration/Sys3 - Power System/Power Generation Subsystem1/Nuclear Power Generation/SP-100 Power Generation/	Power Generation/Sensor Models/Cold Junction Temperature Sensing Model3/ Resistance Temperature Detector/Switch ON//OFF Control Block ==
  In1 : Inport
  In2 : Inport  Port=2
  Multiport Switch1 : MultiPortSwitch  Inputs=2
  Switch OFF : Subsystem
  Switch ON : Subsystem
  Out1 : Outport  VectorParamsAs1DForOutWhenUnconnected=off
  wires:
  Multiport Switch1/1 -> Out1/1
  In2/1 -> Switch ON/1(In1), Switch OFF/1(In1)
  In1/1 -> Multiport Switch1/1
  Switch ON/1(ON) -> Multiport Switch1/2
  Switch OFF/1(OFF) -> Multiport Switch1/3

== system MCVT_Integration/Sys3 - Power System/Power Generation Subsystem1/Nuclear Power Generation/SP-100 Power Generation/	Power Generation/Sensor Models/Cold Junction Temperature Sensing Model4/ Resistance Temperature Detector/RTD with Self-heat Error ==
  Resistance Change with Error : Inport
  " Current in Circuit" : Constant  Value=0.001
  MATLAB Function : MATLAB Function  TreatAsAtomicUnit=on
  Voltage Output with Error : Outport  VectorParamsAs1DForOutWhenUnconnected=off
  wires:
  " Current in Circuit"/1 -> MATLAB Function/1(I)
  MATLAB Function/1(U) -> Voltage Output with Error/1
  Resistance Change with Error/1 -> MATLAB Function/2(R)

== system MCVT_Integration/Sys3 - Power System/Power Generation Subsystem1/Nuclear Power Generation/SP-100 Power Generation/	Power Generation/Sensor Models/Cold Junction Temperature Sensing Model4/ Resistance Temperature Detector/RTD without Self-heat Error ==
  Resistance Change without Error : Inport
  " Current in Circuit" : Constant  Value=0.001
  MATLAB Function : MATLAB Function  TreatAsAtomicUnit=on
  Current in Circuit : Outport  VectorParamsAs1DForOutWhenUnconnected=off
  Voltage Output without Error : Outport  Port=2  VectorParamsAs1DForOutWhenUnconnected=off
  wires:
  " Current in Circuit"/1 -> MATLAB Function/1("I ")
  MATLAB Function/1(I) -> Current in Circuit/1
  MATLAB Function/2(U) -> Voltage Output without Error/1
  Resistance Change without Error/1 -> MATLAB Function/2(R)

== system MCVT_Integration/Sys3 - Power System/Power Generation Subsystem1/Nuclear Power Generation/SP-100 Power Generation/	Power Generation/Sensor Models/Cold Junction Temperature Sensing Model4/ Resistance Temperature Detector/Self-heat Calculation ==
  note: Power = I 2 *R
  Real Temperature : Inport
  Resistance Change without Error : Inport  Port=2
  Current in Circuit : Inport  Port=3
  "  T vs R " : MATLAB Function  TreatAsAtomicUnit=on
  Add1 : Sum
  Divide1 : Product  Inputs=*/
  Gain : Gain  Gain=1000
  Product : Product
  Self Heating Constant (mW/℃) : Constant  Value=15
  Square : Math  Operator=square  SignedPower=on  SaturateOnIntegerOverflow=on
  Resistance Change with Error : Outport  VectorParamsAs1DForOutWhenUnconnected=off
  Self-heat Error : Outport  Port=2  VectorParamsAs1DForOutWhenUnconnected=off
  wires:
  Current in Circuit/1 -> Gain/1
  Square/1 -> Product/2
  Gain/1 -> Square/1
  Real Temperature/1 -> Add1/1
  "  T vs R "/1(R) -> Resistance Change with Error/1
  Resistance Change without Error/1 -> Product/1
  Divide1/1 -> Add1/2, Self-heat Error/1
  Add1/1 -> "  T vs R "/1(T)
  Product/1 -> Divide1/1
  Self Heating Constant (mW/℃)/1 -> Divide1/2

== system MCVT_Integration/Sys3 - Power System/Power Generation Subsystem1/Nuclear Power Generation/SP-100 Power Generation/	Power Generation/Sensor Models/Cold Junction Temperature Sensing Model4/ Resistance Temperature Detector/Switch ON//OFF Control Block ==
  In1 : Inport
  In2 : Inport  Port=2
  Multiport Switch1 : MultiPortSwitch  Inputs=2
  Switch OFF : Subsystem
  Switch ON : Subsystem
  Out1 : Outport  VectorParamsAs1DForOutWhenUnconnected=off
  wires:
  Switch OFF/1(OFF) -> Multiport Switch1/3
  Switch ON/1(ON) -> Multiport Switch1/2
  In1/1 -> Multiport Switch1/1
  In2/1 -> Switch OFF/1(In1), Switch ON/1(In1)
  Multiport Switch1/1 -> Out1/1

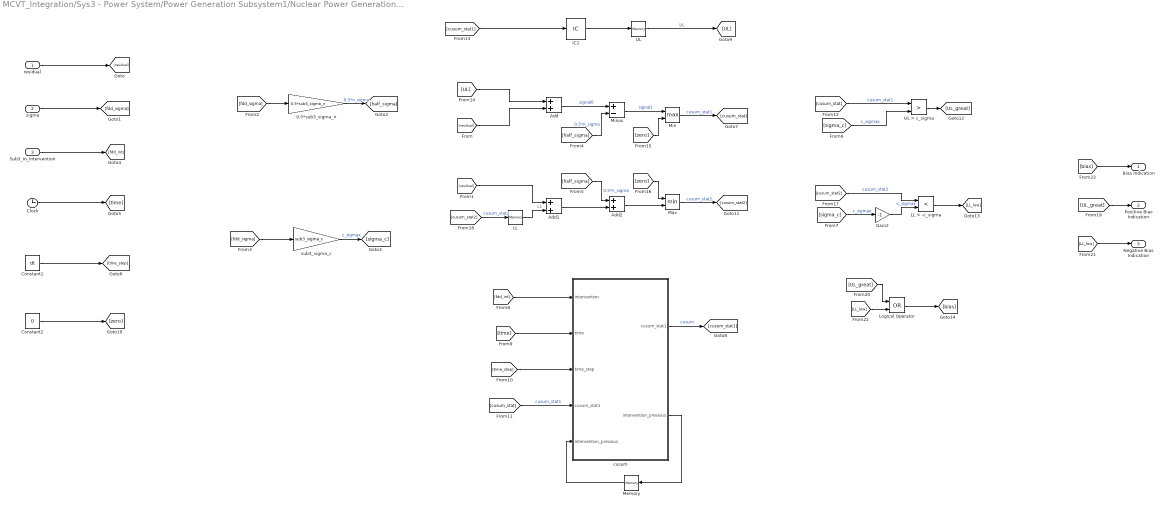
== system MCVT_Integration/Sys3 - Power System/Power Generation Subsystem1/Nuclear Power Generation/SP-100 Power Generation/FDD Iterative Online Residual Analysis1/ Digital Twin of SP100/Residual Generation/Compare to Nominal/CUSUM5 ==
  residual : Inport
  sigma : Inport  Port=2
  Sub3_In_Intervention : Inport  Port=3
  0.5*sub3_sigma_n : Gain  Gain=0.5*sub3_sigma_n
  Add : Sum
  Add1 : Sum
  Add2 : Sum
  Clock : Clock
  Constant1 : Constant  Value=dt
  Constant2 : Constant  Value=0
  Gain2 : Gain  Gain=-1
  IC1 : InitialCondition  Value=0
  LL : Memory
  LL < -c_sigma : RelationalOperator  Operator=<  OutDataTypeStr=boolean  RndMeth=Simplest
  Logical Operator : Logic  Operator=OR  AllPortsSameDT=off  OutDataTypeStr=boolean
  Max : MinMax  Inputs=2
  Memory : Memory
  Min : MinMax  Function=max  Inputs=2
  Minus : Sum  Inputs=+-
  UL : Memory
  UL > c_sigma : RelationalOperator  Operator=>  OutDataTypeStr=boolean  RndMeth=Simplest
  cusum : MATLAB Function  TreatAsAtomicUnit=on
  sub3_sigma_c : Gain  Gain=sub3_sigma_c
  Bias Indication : Outport
  Positive Bias Indication : Outport  Port=2
  Negative Bias Indication : Outport  Port=3
  wires:
  LL/1 -> Add1/2  "LL"
  UL/1 -> [UL]  "UL"
  Add/1 -> Minus/1  "signal0"
  Add1/1 -> Add2/2
  Add2/1 -> Max/2
  Minus/1 -> Min/1  "signal1"
  [zero] -> Min/2
  [cusum_stat] -> cusum/4("cusum_stat1 ")  "cusum_stat1"
  [cusum_stat2] -> LL < -c_sigma/1  "cusum_stat2"
  [half_sigma] -> Minus/2  "0.5*n_sigma"
  [sigma_c] -> UL > c_sigma/2  "c_sigmax"
  [UL_great] -> Positive Bias Indication/1
  [LL_low] -> Negative Bias Indication/1
  Logical Operator/1 -> [bias]
  Gain2/1 -> LL < -c_sigma/2  "-c_sigmax"
  [fdd_sigma] -> 0.5*sub3_sigma_n/1
  [residual] -> Add/2
  cusum/1(cusum_stat1) -> [cusum_stat1]  "cusum"
  [time] -> cusum/2(time)
  [time_step] -> cusum/3(time_step)
  IC1/1 -> UL/1
  [fdd_int] -> cusum/1(intervention)
  cusum/2(intervention_previous) -> Memory/1
  Memory/1 -> cusum/5("intervention_previous ")
  residual/1 -> [residual]
  [residual] -> Add1/1
  sigma/1 -> [fdd_sigma]
  [fdd_sigma] -> sub3_sigma_c/1
  0.5*sub3_sigma_n/1 -> [half_sigma]  "0.5*n_sigma"
  [half_sigma] -> Add2/1  "0.5*n_sigma"
  sub3_sigma_c/1 -> [sigma_c]  "c_sigmax"
  [sigma_c] -> Gain2/1  "c_sigmax"
  Sub3_In_Intervention/1 -> [fdd_int]
  Clock/1 -> [time]
  Constant1/1 -> [time_step]
  Min/1 -> [cusum_stat]  "cusum_stat1"
  [cusum_stat] -> UL > c_sigma/1  "cusum_stat1"
  [cusum_stat1] -> IC1/1
  [UL] -> Add/1
  Constant2/1 -> [zero]
  [zero] -> Max/1
  Max/1 -> [cusum_stat2]  "cusum_stat2"
  [cusum_stat2] -> LL/1  "cusum_stat2"
  UL > c_sigma/1 -> [UL_great]
  [UL_great] -> Logical Operator/1
  LL < -c_sigma/1 -> [LL_low]
  [LL_low] -> Logical Operator/2
  [bias] -> Bias Indication/1

== system MCVT_Integration/Sys3 - Power System/Power Generation Subsystem1/Nuclear Power Generation/SP-100 Power Generation/	Power Generation/Nominal Power/Sensor Models/Cold Junction Temperature Sensing Model/ Resistance Temperature Detector/RTD with Self-heat Error ==
  Resistance Change with Error : Inport
  " Current in Circuit" : Constant  Value=0.001
  MATLAB Function : MATLAB Function  TreatAsAtomicUnit=on
  Voltage Output with Error : Outport  VectorParamsAs1DForOutWhenUnconnected=off
  wires:
  Resistance Change with Error/1 -> MATLAB Function/2(R)
  MATLAB Function/1(U) -> Voltage Output with Error/1
  " Current in Circuit"/1 -> MATLAB Function/1(I)

== system MCVT_Integration/Sys3 - Power System/Power Generation Subsystem1/Nuclear Power Generation/SP-100 Power Generation/	Power Generation/Nominal Power/Sensor Models/Cold Junction Temperature Sensing Model/ Resistance Temperature Detector/RTD without Self-heat Error ==
  Resistance Change without Error : Inport
  " Current in Circuit" : Constant  Value=0.001
  MATLAB Function : MATLAB Function  TreatAsAtomicUnit=on
  Current in Circuit : Outport  VectorParamsAs1DForOutWhenUnconnected=off
  Voltage Output without Error : Outport  Port=2  VectorParamsAs1DForOutWhenUnconnected=off
  wires:
  Resistance Change without Error/1 -> MATLAB Function/2(R)
  MATLAB Function/2(U) -> Voltage Output without Error/1
  MATLAB Function/1(I) -> Current in Circuit/1
  " Current in Circuit"/1 -> MATLAB Function/1("I ")

== system MCVT_Integration/Sys3 - Power System/Power Generation Subsystem1/Nuclear Power Generation/SP-100 Power Generation/	Power Generation/Nominal Power/Sensor Models/Cold Junction Temperature Sensing Model/ Resistance Temperature Detector/Self-heat Calculation ==
  note: Power = I 2 *R
  Real Temperature : Inport
  Resistance Change without Error : Inport  Port=2
  Current in Circuit : Inport  Port=3
  "  T vs R " : MATLAB Function  TreatAsAtomicUnit=on
  Add1 : Sum
  Divide1 : Product  Inputs=*/
  Gain : Gain  Gain=1000
  Product : Product
  Self Heating Constant (mW/℃) : Constant  Value=15
  Square : Math  Operator=square  SignedPower=on  SaturateOnIntegerOverflow=on
  Resistance Change with Error : Outport  VectorParamsAs1DForOutWhenUnconnected=off
  Self-heat Error : Outport  Port=2  VectorParamsAs1DForOutWhenUnconnected=off
  wires:
  Self Heating Constant (mW/℃)/1 -> Divide1/2
  Product/1 -> Divide1/1
  Add1/1 -> "  T vs R "/1(T)
  Divide1/1 -> Self-heat Error/1, Add1/2
  Resistance Change without Error/1 -> Product/1
  "  T vs R "/1(R) -> Resistance Change with Error/1
  Real Temperature/1 -> Add1/1
  Gain/1 -> Square/1
  Square/1 -> Product/2
  Current in Circuit/1 -> Gain/1
